simulink model "cddDcmApp"  (R2022b)
settings: Solver=FixedStepDiscrete  StartTime=0.0  StopTime=10.0  FixedStep=0.02  RelTol=1e-3
undefined symbols (not defined in the file or in its folder): CCPFaultType, DcChlType, HFAChlType, IcuChannelType, MtrRoutStaEnum, P_SignalPartType, P_Std_ReturnType, PowerChlType, SrsAdChlType, VGAChlType
(part 11 of 13)

== system cddDcmApp/DataServices_DID_D970_Stepper_Motor_12_Mode_Panel_First_Left_Flap_DID_D970_1_step_Range/DataServices_DID_D970_Stepper_Motor_12_Mode_Panel_First_Left_Flap_DID_D970_1_step_Range_WriteData_sys ==
  function : TriggerPort  TriggerType=function-call  IsSimulinkFunction=on  FunctionName=DataServices_DID_D970_Stepper_Motor_12_Mode_Panel_First_Left_Flap_DID_D970_1_step_Range_WriteData  VariantControl=(inherit)
  Ground_1 : Ground
  Ground_2 : Ground
  Terminator_1 : Terminator
  Terminator_2 : Terminator
  Data : ArgIn  OutDataTypeStr=uint8  PortDimensions=2  ArgumentName=Data
  OpStatus : ArgIn  Port=2  OutDataTypeStr=Enum: Dcm_OpStatusType  ArgumentName=OpStatus
  ErrorCode : ArgOut  OutDataTypeStr=Enum: Dcm_NegativeResponseCodeType  ArgumentName=ErrorCode
  ERR : ArgOut  Port=2  OutDataTypeStr=Std_ReturnType  ArgumentName=ERR
  wires:
  Data/1 -> Terminator_1/1
  OpStatus/1 -> Terminator_2/1
  Ground_1/1 -> ErrorCode/1
  Ground_2/1 -> ERR/1

== system cddDcmApp/DataServices_DID_D970_Stepper_Motor_12_Mode_Panel_First_Left_Flap_DID_D970_2_No_Of_Movements/DataServices_DID_D970_Stepper_Motor_12_Mode_Panel_First_Left_Flap_DID_D970_2_No_Of_Movements_ConditionCheckRead_sys ==
  function : TriggerPort  TriggerType=function-call  IsSimulinkFunction=on  FunctionName=DataServices_DID_D970_Stepper_Motor_12_Mode_Panel_First_Left_Flap_DID_D970_2_No_Of_Movements_ConditionCheckRead  VariantControl=(inherit)
  Ground_1 : Ground
  Ground_2 : Ground
  ErrorCode : ArgOut  OutDataTypeStr=Enum: Dcm_NegativeResponseCodeType  ArgumentName=ErrorCode
  ERR : ArgOut  Port=2  OutDataTypeStr=Std_ReturnType  ArgumentName=ERR
  wires:
  Ground_1/1 -> ErrorCode/1
  Ground_2/1 -> ERR/1

== system cddDcmApp/DataServices_DID_D970_Stepper_Motor_12_Mode_Panel_First_Left_Flap_DID_D970_2_No_Of_Movements/DataServices_DID_D970_Stepper_Motor_12_Mode_Panel_First_Left_Flap_DID_D970_2_No_Of_Movements_ReadData_sys ==
  function : TriggerPort  TriggerType=function-call  IsSimulinkFunction=on  FunctionName=DataServices_DID_D970_Stepper_Motor_12_Mode_Panel_First_Left_Flap_DID_D970_2_No_Of_Movements_ReadData  VariantControl=(inherit)
  Ground_1 : Ground
  Ground_2 : Ground
  Data : ArgOut  OutDataTypeStr=uint8  ArgumentName=Data
  ERR : ArgOut  Port=2  OutDataTypeStr=Std_ReturnType  ArgumentName=ERR
  wires:
  Ground_1/1 -> Data/1
  Ground_2/1 -> ERR/1

== system cddDcmApp/DataServices_DID_D970_Stepper_Motor_12_Mode_Panel_First_Left_Flap_DID_D970_2_No_Of_Movements/DataServices_DID_D970_Stepper_Motor_12_Mode_Panel_First_Left_Flap_DID_D970_2_No_Of_Movements_WriteData_sys ==
  function : TriggerPort  TriggerType=function-call  IsSimulinkFunction=on  FunctionName=DataServices_DID_D970_Stepper_Motor_12_Mode_Panel_First_Left_Flap_DID_D970_2_No_Of_Movements_WriteData  VariantControl=(inherit)
  Ground_1 : Ground
  Ground_2 : Ground
  Terminator_1 : Terminator
  Terminator_2 : Terminator
  Data : ArgIn  OutDataTypeStr=uint8  PortDimensions=4  ArgumentName=Data
  OpStatus : ArgIn  Port=2  OutDataTypeStr=Enum: Dcm_OpStatusType  ArgumentName=OpStatus
  ErrorCode : ArgOut  OutDataTypeStr=Enum: Dcm_NegativeResponseCodeType  ArgumentName=ErrorCode
  ERR : ArgOut  Port=2  OutDataTypeStr=Std_ReturnType  ArgumentName=ERR
  wires:
  Data/1 -> Terminator_1/1
  OpStatus/1 -> Terminator_2/1
  Ground_1/1 -> ErrorCode/1
  Ground_2/1 -> ERR/1

== system cddDcmApp/DataServices_DID_D971_Stepper_Motor_12_Mode_Panel_First_Left_Flap_position_ComplexStructure_2/DataServices_DID_D971_Stepper_Motor_12_Mode_Panel_First_Left_Flap_position_ComplexStructure_2_ConditionCheckRead_sys ==
  function : TriggerPort  TriggerType=function-call  IsSimulinkFunction=on  FunctionName=DataServices_DID_D971_Stepper_Motor_12_Mode_Panel_First_Left_Flap_position_ComplexStructure_2_ConditionCheckRead  VariantControl=(inherit)
  Ground_1 : Ground
  Ground_2 : Ground
  ErrorCode : ArgOut  OutDataTypeStr=Enum: Dcm_NegativeResponseCodeType  ArgumentName=ErrorCode
  ERR : ArgOut  Port=2  OutDataTypeStr=Std_ReturnType  ArgumentName=ERR
  wires:
  Ground_1/1 -> ErrorCode/1
  Ground_2/1 -> ERR/1

== system cddDcmApp/DataServices_DID_D971_Stepper_Motor_12_Mode_Panel_First_Left_Flap_position_ComplexStructure_2/DataServices_DID_D971_Stepper_Motor_12_Mode_Panel_First_Left_Flap_position_ComplexStructure_2_ReadData_sys ==
  function : TriggerPort  TriggerType=function-call  IsSimulinkFunction=on  FunctionName=DataServices_DID_D971_Stepper_Motor_12_Mode_Panel_First_Left_Flap_position_ComplexStructure_2_ReadData  VariantControl=(inherit)
  Ground_1 : Ground
  Ground_2 : Ground
  Data : ArgOut  OutDataTypeStr=uint8  ArgumentName=Data
  ERR : ArgOut  Port=2  OutDataTypeStr=Std_ReturnType  ArgumentName=ERR
  wires:
  Ground_1/1 -> Data/1
  Ground_2/1 -> ERR/1

== system cddDcmApp/DataServices_DID_D971_Stepper_Motor_12_Mode_Panel_First_Left_Flap_position_DID_D971_1_Requested_Position/DataServices_DID_D971_Stepper_Motor_12_Mode_Panel_First_Left_Flap_position_DID_D971_1_Requested_Position_ConditionCheckRead_sys ==
  function : TriggerPort  TriggerType=function-call  IsSimulinkFunction=on  FunctionName=<binary blob, 123 chars, not shown>  VariantControl=(inherit)
  Ground_1 : Ground
  Ground_2 : Ground
  ErrorCode : ArgOut  OutDataTypeStr=Enum: Dcm_NegativeResponseCodeType  ArgumentName=ErrorCode
  ERR : ArgOut  Port=2  OutDataTypeStr=Std_ReturnType  ArgumentName=ERR
  wires:
  Ground_1/1 -> ErrorCode/1
  Ground_2/1 -> ERR/1

== system cddDcmApp/DataServices_DID_D971_Stepper_Motor_12_Mode_Panel_First_Left_Flap_position_DID_D971_1_Requested_Position/DataServices_DID_D971_Stepper_Motor_12_Mode_Panel_First_Left_Flap_position_DID_D971_1_Requested_Position_ReadData_sys ==
  function : TriggerPort  TriggerType=function-call  IsSimulinkFunction=on  FunctionName=DataServices_DID_D971_Stepper_Motor_12_Mode_Panel_First_Left_Flap_position_DID_D971_1_Requested_Position_ReadData  VariantControl=(inherit)
  Ground_1 : Ground
  Ground_2 : Ground
  Data : ArgOut  OutDataTypeStr=uint8  ArgumentName=Data
  ERR : ArgOut  Port=2  OutDataTypeStr=Std_ReturnType  ArgumentName=ERR
  wires:
  Ground_1/1 -> Data/1
  Ground_2/1 -> ERR/1

== system cddDcmApp/DataServices_DID_D971_Stepper_Motor_12_Mode_Panel_First_Left_Flap_position_DID_D971_2_Actual_Position/DataServices_DID_D971_Stepper_Motor_12_Mode_Panel_First_Left_Flap_position_DID_D971_2_Actual_Position_ConditionCheckRead_sys ==
  function : TriggerPort  TriggerType=function-call  IsSimulinkFunction=on  FunctionName=<binary blob, 120 chars, not shown>  VariantControl=(inherit)
  Ground_1 : Ground
  Ground_2 : Ground
  ErrorCode : ArgOut  OutDataTypeStr=Enum: Dcm_NegativeResponseCodeType  ArgumentName=ErrorCode
  ERR : ArgOut  Port=2  OutDataTypeStr=Std_ReturnType  ArgumentName=ERR
  wires:
  Ground_1/1 -> ErrorCode/1
  Ground_2/1 -> ERR/1

== system cddDcmApp/DataServices_DID_D971_Stepper_Motor_12_Mode_Panel_First_Left_Flap_position_DID_D971_2_Actual_Position/DataServices_DID_D971_Stepper_Motor_12_Mode_Panel_First_Left_Flap_position_DID_D971_2_Actual_Position_ReadData_sys ==
  function : TriggerPort  TriggerType=function-call  IsSimulinkFunction=on  FunctionName=DataServices_DID_D971_Stepper_Motor_12_Mode_Panel_First_Left_Flap_position_DID_D971_2_Actual_Position_ReadData  VariantControl=(inherit)
  Ground_1 : Ground
  Ground_2 : Ground
  Data : ArgOut  OutDataTypeStr=uint8  ArgumentName=Data
  ERR : ArgOut  Port=2  OutDataTypeStr=Std_ReturnType  ArgumentName=ERR
  wires:
  Ground_1/1 -> Data/1
  Ground_2/1 -> ERR/1

== system cddDcmApp/DataServices_DID_D972_Stepper_Motor_13_Mode_Panel_First_Side_Left_Flap_DID_D972_1_step_Range/DataServices_DID_D972_Stepper_Motor_13_Mode_Panel_First_Side_Left_Flap_DID_D972_1_step_Range_ConditionCheckRead_sys ==
  function : TriggerPort  TriggerType=function-call  IsSimulinkFunction=on  FunctionName=DataServices_DID_D972_Stepper_Motor_13_Mode_Panel_First_Side_Left_Flap_DID_D972_1_step_Range_ConditionCheckRead  VariantControl=(inherit)
  Ground_1 : Ground
  Ground_2 : Ground
  ErrorCode : ArgOut  OutDataTypeStr=Enum: Dcm_NegativeResponseCodeType  ArgumentName=ErrorCode
  ERR : ArgOut  Port=2  OutDataTypeStr=Std_ReturnType  ArgumentName=ERR
  wires:
  Ground_1/1 -> ErrorCode/1
  Ground_2/1 -> ERR/1

== system cddDcmApp/DataServices_DID_D972_Stepper_Motor_13_Mode_Panel_First_Side_Left_Flap_DID_D972_1_step_Range/DataServices_DID_D972_Stepper_Motor_13_Mode_Panel_First_Side_Left_Flap_DID_D972_1_step_Range_ReadData_sys ==
  function : TriggerPort  TriggerType=function-call  IsSimulinkFunction=on  FunctionName=DataServices_DID_D972_Stepper_Motor_13_Mode_Panel_First_Side_Left_Flap_DID_D972_1_step_Range_ReadData  VariantControl=(inherit)
  Ground_1 : Ground
  Ground_2 : Ground
  Data : ArgOut  OutDataTypeStr=uint8  ArgumentName=Data
  ERR : ArgOut  Port=2  OutDataTypeStr=Std_ReturnType  ArgumentName=ERR
  wires:
  Ground_1/1 -> Data/1
  Ground_2/1 -> ERR/1

== system cddDcmApp/DataServices_DID_D972_Stepper_Motor_13_Mode_Panel_First_Side_Left_Flap_DID_D972_1_step_Range/DataServices_DID_D972_Stepper_Motor_13_Mode_Panel_First_Side_Left_Flap_DID_D972_1_step_Range_WriteData_sys ==
  function : TriggerPort  TriggerType=function-call  IsSimulinkFunction=on  FunctionName=DataServices_DID_D972_Stepper_Motor_13_Mode_Panel_First_Side_Left_Flap_DID_D972_1_step_Range_WriteData  VariantControl=(inherit)
  Ground_1 : Ground
  Ground_2 : Ground
  Terminator_1 : Terminator
  Terminator_2 : Terminator
  Data : ArgIn  OutDataTypeStr=uint8  PortDimensions=2  ArgumentName=Data
  OpStatus : ArgIn  Port=2  OutDataTypeStr=Enum: Dcm_OpStatusType  ArgumentName=OpStatus
  ErrorCode : ArgOut  OutDataTypeStr=Enum: Dcm_NegativeResponseCodeType  ArgumentName=ErrorCode
  ERR : ArgOut  Port=2  OutDataTypeStr=Std_ReturnType  ArgumentName=ERR
  wires:
  Data/1 -> Terminator_1/1
  OpStatus/1 -> Terminator_2/1
  Ground_1/1 -> ErrorCode/1
  Ground_2/1 -> ERR/1

== system cddDcmApp/DataServices_DID_D972_Stepper_Motor_13_Mode_Panel_First_Side_Left_Flap_DID_D972_2_No_Of_Movements/DataServices_DID_D972_Stepper_Motor_13_Mode_Panel_First_Side_Left_Flap_DID_D972_2_No_Of_Movements_ConditionCheckRead_sys ==
  function : TriggerPort  TriggerType=function-call  IsSimulinkFunction=on  FunctionName=DataServices_DID_D972_Stepper_Motor_13_Mode_Panel_First_Side_Left_Flap_DID_D972_2_No_Of_Movements_ConditionCheckRead  VariantControl=(inherit)
  Ground_1 : Ground
  Ground_2 : Ground
  ErrorCode : ArgOut  OutDataTypeStr=Enum: Dcm_NegativeResponseCodeType  ArgumentName=ErrorCode
  ERR : ArgOut  Port=2  OutDataTypeStr=Std_ReturnType  ArgumentName=ERR
  wires:
  Ground_1/1 -> ErrorCode/1
  Ground_2/1 -> ERR/1

== system cddDcmApp/DataServices_DID_D972_Stepper_Motor_13_Mode_Panel_First_Side_Left_Flap_DID_D972_2_No_Of_Movements/DataServices_DID_D972_Stepper_Motor_13_Mode_Panel_First_Side_Left_Flap_DID_D972_2_No_Of_Movements_ReadData_sys ==
  function : TriggerPort  TriggerType=function-call  IsSimulinkFunction=on  FunctionName=DataServices_DID_D972_Stepper_Motor_13_Mode_Panel_First_Side_Left_Flap_DID_D972_2_No_Of_Movements_ReadData  VariantControl=(inherit)
  Ground_1 : Ground
  Ground_2 : Ground
  Data : ArgOut  OutDataTypeStr=uint8  ArgumentName=Data
  ERR : ArgOut  Port=2  OutDataTypeStr=Std_ReturnType  ArgumentName=ERR
  wires:
  Ground_1/1 -> Data/1
  Ground_2/1 -> ERR/1

== system cddDcmApp/DataServices_DID_D972_Stepper_Motor_13_Mode_Panel_First_Side_Left_Flap_DID_D972_2_No_Of_Movements/DataServices_DID_D972_Stepper_Motor_13_Mode_Panel_First_Side_Left_Flap_DID_D972_2_No_Of_Movements_WriteData_sys ==
  function : TriggerPort  TriggerType=function-call  IsSimulinkFunction=on  FunctionName=DataServices_DID_D972_Stepper_Motor_13_Mode_Panel_First_Side_Left_Flap_DID_D972_2_No_Of_Movements_WriteData  VariantControl=(inherit)
  Ground_1 : Ground
  Ground_2 : Ground
  Terminator_1 : Terminator
  Terminator_2 : Terminator
  Data : ArgIn  OutDataTypeStr=uint8  PortDimensions=4  ArgumentName=Data
  OpStatus : ArgIn  Port=2  OutDataTypeStr=Enum: Dcm_OpStatusType  ArgumentName=OpStatus
  ErrorCode : ArgOut  OutDataTypeStr=Enum: Dcm_NegativeResponseCodeType  ArgumentName=ErrorCode
  ERR : ArgOut  Port=2  OutDataTypeStr=Std_ReturnType  ArgumentName=ERR
  wires:
  Data/1 -> Terminator_1/1
  OpStatus/1 -> Terminator_2/1
  Ground_1/1 -> ErrorCode/1
  Ground_2/1 -> ERR/1

== system cddDcmApp/DataServices_DID_D973_Stepper_Motor_13_Mode_Panel_First_Side_Left_Flap_position_ComplexStructure_2/DataServices_DID_D973_Stepper_Motor_13_Mode_Panel_First_Side_Left_Flap_position_ComplexStructure_2_ConditionCheckRead_sys ==
  function : TriggerPort  TriggerType=function-call  IsSimulinkFunction=on  FunctionName=DataServices_DID_D973_Stepper_Motor_13_Mode_Panel_First_Side_Left_Flap_position_ComplexStructure_2_ConditionCheckRead  VariantControl=(inherit)
  Ground_1 : Ground
  Ground_2 : Ground
  ErrorCode : ArgOut  OutDataTypeStr=Enum: Dcm_NegativeResponseCodeType  ArgumentName=ErrorCode
  ERR : ArgOut  Port=2  OutDataTypeStr=Std_ReturnType  ArgumentName=ERR
  wires:
  Ground_1/1 -> ErrorCode/1
  Ground_2/1 -> ERR/1

== system cddDcmApp/DataServices_DID_D973_Stepper_Motor_13_Mode_Panel_First_Side_Left_Flap_position_ComplexStructure_2/DataServices_DID_D973_Stepper_Motor_13_Mode_Panel_First_Side_Left_Flap_position_ComplexStructure_2_ReadData_sys ==
  function : TriggerPort  TriggerType=function-call  IsSimulinkFunction=on  FunctionName=DataServices_DID_D973_Stepper_Motor_13_Mode_Panel_First_Side_Left_Flap_position_ComplexStructure_2_ReadData  VariantControl=(inherit)
  Ground_1 : Ground
  Ground_2 : Ground
  Data : ArgOut  OutDataTypeStr=uint8  ArgumentName=Data
  ERR : ArgOut  Port=2  OutDataTypeStr=Std_ReturnType  ArgumentName=ERR
  wires:
  Ground_1/1 -> Data/1
  Ground_2/1 -> ERR/1

== system cddDcmApp/DataServices_DID_D973_Stepper_Motor_13_Mode_Panel_First_Side_Left_Flap_position_DID_D973_1_Requested_Position/DataServices_DID_D973_Stepper_Motor_13_Mode_Panel_First_Side_Left_Flap_position_DID_D973_1_Requested_Position_ConditionCheckRead_sys ==
  function : TriggerPort  TriggerType=function-call  IsSimulinkFunction=on  FunctionName=<binary blob, 128 chars, not shown>  VariantControl=(inherit)
  Ground_1 : Ground
  Ground_2 : Ground
  ErrorCode : ArgOut  OutDataTypeStr=Enum: Dcm_NegativeResponseCodeType  ArgumentName=ErrorCode
  ERR : ArgOut  Port=2  OutDataTypeStr=Std_ReturnType  ArgumentName=ERR
  wires:
  Ground_1/1 -> ErrorCode/1
  Ground_2/1 -> ERR/1

== system cddDcmApp/DataServices_DID_D973_Stepper_Motor_13_Mode_Panel_First_Side_Left_Flap_position_DID_D973_1_Requested_Position/DataServices_DID_D973_Stepper_Motor_13_Mode_Panel_First_Side_Left_Flap_position_DID_D973_1_Requested_Position_ReadData_sys ==
  function : TriggerPort  TriggerType=function-call  IsSimulinkFunction=on  FunctionName=DataServices_DID_D973_Stepper_Motor_13_Mode_Panel_First_Side_Left_Flap_position_DID_D973_1_Requested_Position_ReadData  VariantControl=(inherit)
  Ground_1 : Ground
  Ground_2 : Ground
  Data : ArgOut  OutDataTypeStr=uint8  ArgumentName=Data
  ERR : ArgOut  Port=2  OutDataTypeStr=Std_ReturnType  ArgumentName=ERR
  wires:
  Ground_1/1 -> Data/1
  Ground_2/1 -> ERR/1

== system cddDcmApp/DataServices_DID_D973_Stepper_Motor_13_Mode_Panel_First_Side_Left_Flap_position_DID_D973_2_Actual_Position/DataServices_DID_D973_Stepper_Motor_13_Mode_Panel_First_Side_Left_Flap_position_DID_D973_2_Actual_Position_ConditionCheckRead_sys ==
  function : TriggerPort  TriggerType=function-call  IsSimulinkFunction=on  FunctionName=<binary blob, 125 chars, not shown>  VariantControl=(inherit)
  Ground_1 : Ground
  Ground_2 : Ground
  ErrorCode : ArgOut  OutDataTypeStr=Enum: Dcm_NegativeResponseCodeType  ArgumentName=ErrorCode
  ERR : ArgOut  Port=2  OutDataTypeStr=Std_ReturnType  ArgumentName=ERR
  wires:
  Ground_1/1 -> ErrorCode/1
  Ground_2/1 -> ERR/1

== system cddDcmApp/DataServices_DID_D973_Stepper_Motor_13_Mode_Panel_First_Side_Left_Flap_position_DID_D973_2_Actual_Position/DataServices_DID_D973_Stepper_Motor_13_Mode_Panel_First_Side_Left_Flap_position_DID_D973_2_Actual_Position_ReadData_sys ==
  function : TriggerPort  TriggerType=function-call  IsSimulinkFunction=on  FunctionName=DataServices_DID_D973_Stepper_Motor_13_Mode_Panel_First_Side_Left_Flap_position_DID_D973_2_Actual_Position_ReadData  VariantControl=(inherit)
  Ground_1 : Ground
  Ground_2 : Ground
  Data : ArgOut  OutDataTypeStr=uint8  ArgumentName=Data
  ERR : ArgOut  Port=2  OutDataTypeStr=Std_ReturnType  ArgumentName=ERR
  wires:
  Ground_1/1 -> Data/1
  Ground_2/1 -> ERR/1

== system cddDcmApp/DataServices_DID_D974_Stepper_Motor_14_Mode_Panel_First_Right_Flap_DID_D974_1_step_Range/DataServices_DID_D974_Stepper_Motor_14_Mode_Panel_First_Right_Flap_DID_D974_1_step_Range_ConditionCheckRead_sys ==
  function : TriggerPort  TriggerType=function-call  IsSimulinkFunction=on  FunctionName=DataServices_DID_D974_Stepper_Motor_14_Mode_Panel_First_Right_Flap_DID_D974_1_step_Range_ConditionCheckRead  VariantControl=(inherit)
  Ground_1 : Ground
  Ground_2 : Ground
  ErrorCode : ArgOut  OutDataTypeStr=Enum: Dcm_NegativeResponseCodeType  ArgumentName=ErrorCode
  ERR : ArgOut  Port=2  OutDataTypeStr=Std_ReturnType  ArgumentName=ERR
  wires:
  Ground_1/1 -> ErrorCode/1
  Ground_2/1 -> ERR/1

== system cddDcmApp/DataServices_DID_D974_Stepper_Motor_14_Mode_Panel_First_Right_Flap_DID_D974_1_step_Range/DataServices_DID_D974_Stepper_Motor_14_Mode_Panel_First_Right_Flap_DID_D974_1_step_Range_ReadData_sys ==
  function : TriggerPort  TriggerType=function-call  IsSimulinkFunction=on  FunctionName=DataServices_DID_D974_Stepper_Motor_14_Mode_Panel_First_Right_Flap_DID_D974_1_step_Range_ReadData  VariantControl=(inherit)
  Ground_1 : Ground
  Ground_2 : Ground
  Data : ArgOut  OutDataTypeStr=uint8  ArgumentName=Data
  ERR : ArgOut  Port=2  OutDataTypeStr=Std_ReturnType  ArgumentName=ERR
  wires:
  Ground_1/1 -> Data/1
  Ground_2/1 -> ERR/1

== system cddDcmApp/DataServices_DID_D974_Stepper_Motor_14_Mode_Panel_First_Right_Flap_DID_D974_1_step_Range/DataServices_DID_D974_Stepper_Motor_14_Mode_Panel_First_Right_Flap_DID_D974_1_step_Range_WriteData_sys ==
  function : TriggerPort  TriggerType=function-call  IsSimulinkFunction=on  FunctionName=DataServices_DID_D974_Stepper_Motor_14_Mode_Panel_First_Right_Flap_DID_D974_1_step_Range_WriteData  VariantControl=(inherit)
  Ground_1 : Ground
  Ground_2 : Ground
  Terminator_1 : Terminator
  Terminator_2 : Terminator
  Data : ArgIn  OutDataTypeStr=uint8  PortDimensions=2  ArgumentName=Data
  OpStatus : ArgIn  Port=2  OutDataTypeStr=Enum: Dcm_OpStatusType  ArgumentName=OpStatus
  ErrorCode : ArgOut  OutDataTypeStr=Enum: Dcm_NegativeResponseCodeType  ArgumentName=ErrorCode
  ERR : ArgOut  Port=2  OutDataTypeStr=Std_ReturnType  ArgumentName=ERR
  wires:
  Data/1 -> Terminator_1/1
  OpStatus/1 -> Terminator_2/1
  Ground_1/1 -> ErrorCode/1
  Ground_2/1 -> ERR/1

== system cddDcmApp/DataServices_DID_D974_Stepper_Motor_14_Mode_Panel_First_Right_Flap_DID_D974_2_No_Of_Movements/DataServices_DID_D974_Stepper_Motor_14_Mode_Panel_First_Right_Flap_DID_D974_2_No_Of_Movements_ConditionCheckRead_sys ==
  function : TriggerPort  TriggerType=function-call  IsSimulinkFunction=on  FunctionName=DataServices_DID_D974_Stepper_Motor_14_Mode_Panel_First_Right_Flap_DID_D974_2_No_Of_Movements_ConditionCheckRead  VariantControl=(inherit)
  Ground_1 : Ground
  Ground_2 : Ground
  ErrorCode : ArgOut  OutDataTypeStr=Enum: Dcm_NegativeResponseCodeType  ArgumentName=ErrorCode
  ERR : ArgOut  Port=2  OutDataTypeStr=Std_ReturnType  ArgumentName=ERR
  wires:
  Ground_1/1 -> ErrorCode/1
  Ground_2/1 -> ERR/1

== system cddDcmApp/DataServices_DID_D974_Stepper_Motor_14_Mode_Panel_First_Right_Flap_DID_D974_2_No_Of_Movements/DataServices_DID_D974_Stepper_Motor_14_Mode_Panel_First_Right_Flap_DID_D974_2_No_Of_Movements_ReadData_sys ==
  function : TriggerPort  TriggerType=function-call  IsSimulinkFunction=on  FunctionName=DataServices_DID_D974_Stepper_Motor_14_Mode_Panel_First_Right_Flap_DID_D974_2_No_Of_Movements_ReadData  VariantControl=(inherit)
  Ground_1 : Ground
  Ground_2 : Ground
  Data : ArgOut  OutDataTypeStr=uint8  ArgumentName=Data
  ERR : ArgOut  Port=2  OutDataTypeStr=Std_ReturnType  ArgumentName=ERR
  wires:
  Ground_1/1 -> Data/1
  Ground_2/1 -> ERR/1

== system cddDcmApp/DataServices_DID_D974_Stepper_Motor_14_Mode_Panel_First_Right_Flap_DID_D974_2_No_Of_Movements/DataServices_DID_D974_Stepper_Motor_14_Mode_Panel_First_Right_Flap_DID_D974_2_No_Of_Movements_WriteData_sys ==
  function : TriggerPort  TriggerType=function-call  IsSimulinkFunction=on  FunctionName=DataServices_DID_D974_Stepper_Motor_14_Mode_Panel_First_Right_Flap_DID_D974_2_No_Of_Movements_WriteData  VariantControl=(inherit)
  Ground_1 : Ground
  Ground_2 : Ground
  Terminator_1 : Terminator
  Terminator_2 : Terminator
  Data : ArgIn  OutDataTypeStr=uint8  PortDimensions=4  ArgumentName=Data
  OpStatus : ArgIn  Port=2  OutDataTypeStr=Enum: Dcm_OpStatusType  ArgumentName=OpStatus
  ErrorCode : ArgOut  OutDataTypeStr=Enum: Dcm_NegativeResponseCodeType  ArgumentName=ErrorCode
  ERR : ArgOut  Port=2  OutDataTypeStr=Std_ReturnType  ArgumentName=ERR
  wires:
  Data/1 -> Terminator_1/1
  OpStatus/1 -> Terminator_2/1
  Ground_1/1 -> ErrorCode/1
  Ground_2/1 -> ERR/1

== system cddDcmApp/DataServices_DID_D975_Stepper_Motor_14_Mode_Panel_First_Right_Flap_position_ComplexStructure_2/DataServices_DID_D975_Stepper_Motor_14_Mode_Panel_First_Right_Flap_position_ComplexStructure_2_ConditionCheckRead_sys ==
  function : TriggerPort  TriggerType=function-call  IsSimulinkFunction=on  FunctionName=DataServices_DID_D975_Stepper_Motor_14_Mode_Panel_First_Right_Flap_position_ComplexStructure_2_ConditionCheckRead  VariantControl=(inherit)
  Ground_1 : Ground
  Ground_2 : Ground
  ErrorCode : ArgOut  OutDataTypeStr=Enum: Dcm_NegativeResponseCodeType  ArgumentName=ErrorCode
  ERR : ArgOut  Port=2  OutDataTypeStr=Std_ReturnType  ArgumentName=ERR
  wires:
  Ground_1/1 -> ErrorCode/1
  Ground_2/1 -> ERR/1

== system cddDcmApp/DataServices_DID_D975_Stepper_Motor_14_Mode_Panel_First_Right_Flap_position_ComplexStructure_2/DataServices_DID_D975_Stepper_Motor_14_Mode_Panel_First_Right_Flap_position_ComplexStructure_2_ReadData_sys ==
  function : TriggerPort  TriggerType=function-call  IsSimulinkFunction=on  FunctionName=DataServices_DID_D975_Stepper_Motor_14_Mode_Panel_First_Right_Flap_position_ComplexStructure_2_ReadData  VariantControl=(inherit)
  Ground_1 : Ground
  Ground_2 : Ground
  Data : ArgOut  OutDataTypeStr=uint8  ArgumentName=Data
  ERR : ArgOut  Port=2  OutDataTypeStr=Std_ReturnType  ArgumentName=ERR
  wires:
  Ground_1/1 -> Data/1
  Ground_2/1 -> ERR/1

== system cddDcmApp/DataServices_DID_D975_Stepper_Motor_14_Mode_Panel_First_Right_Flap_position_DID_D975_1_Requested_Position/DataServices_DID_D975_Stepper_Motor_14_Mode_Panel_First_Right_Flap_position_DID_D975_1_Requested_Position_ConditionCheckRead_sys ==
  function : TriggerPort  TriggerType=function-call  IsSimulinkFunction=on  FunctionName=<binary blob, 124 chars, not shown>  VariantControl=(inherit)
  Ground_1 : Ground
  Ground_2 : Ground
  ErrorCode : ArgOut  OutDataTypeStr=Enum: Dcm_NegativeResponseCodeType  ArgumentName=ErrorCode
  ERR : ArgOut  Port=2  OutDataTypeStr=Std_ReturnType  ArgumentName=ERR
  wires:
  Ground_1/1 -> ErrorCode/1
  Ground_2/1 -> ERR/1

== system cddDcmApp/DataServices_DID_D975_Stepper_Motor_14_Mode_Panel_First_Right_Flap_position_DID_D975_1_Requested_Position/DataServices_DID_D975_Stepper_Motor_14_Mode_Panel_First_Right_Flap_position_DID_D975_1_Requested_Position_ReadData_sys ==
  function : TriggerPort  TriggerType=function-call  IsSimulinkFunction=on  FunctionName=DataServices_DID_D975_Stepper_Motor_14_Mode_Panel_First_Right_Flap_position_DID_D975_1_Requested_Position_ReadData  VariantControl=(inherit)
  Ground_1 : Ground
  Ground_2 : Ground
  Data : ArgOut  OutDataTypeStr=uint8  ArgumentName=Data
  ERR : ArgOut  Port=2  OutDataTypeStr=Std_ReturnType  ArgumentName=ERR
  wires:
  Ground_1/1 -> Data/1
  Ground_2/1 -> ERR/1

== system cddDcmApp/DataServices_DID_D975_Stepper_Motor_14_Mode_Panel_First_Right_Flap_position_DID_D975_2_Actual_Position/DataServices_DID_D975_Stepper_Motor_14_Mode_Panel_First_Right_Flap_position_DID_D975_2_Actual_Position_ConditionCheckRead_sys ==
  function : TriggerPort  TriggerType=function-call  IsSimulinkFunction=on  FunctionName=<binary blob, 121 chars, not shown>  VariantControl=(inherit)
  Ground_1 : Ground
  Ground_2 : Ground
  ErrorCode : ArgOut  OutDataTypeStr=Enum: Dcm_NegativeResponseCodeType  ArgumentName=ErrorCode
  ERR : ArgOut  Port=2  OutDataTypeStr=Std_ReturnType  ArgumentName=ERR
  wires:
  Ground_1/1 -> ErrorCode/1
  Ground_2/1 -> ERR/1

== system cddDcmApp/DataServices_DID_D975_Stepper_Motor_14_Mode_Panel_First_Right_Flap_position_DID_D975_2_Actual_Position/DataServices_DID_D975_Stepper_Motor_14_Mode_Panel_First_Right_Flap_position_DID_D975_2_Actual_Position_ReadData_sys ==
  function : TriggerPort  TriggerType=function-call  IsSimulinkFunction=on  FunctionName=DataServices_DID_D975_Stepper_Motor_14_Mode_Panel_First_Right_Flap_position_DID_D975_2_Actual_Position_ReadData  VariantControl=(inherit)
  Ground_1 : Ground
  Ground_2 : Ground
  Data : ArgOut  OutDataTypeStr=uint8  ArgumentName=Data
  ERR : ArgOut  Port=2  OutDataTypeStr=Std_ReturnType  ArgumentName=ERR
  wires:
  Ground_1/1 -> Data/1
  Ground_2/1 -> ERR/1

== system cddDcmApp/DataServices_DID_D976_Stepper_Motor_15_Mode_Panel_First_Side_Right_Flap_DID_D976_1_step_Range/DataServices_DID_D976_Stepper_Motor_15_Mode_Panel_First_Side_Right_Flap_DID_D976_1_step_Range_ConditionCheckRead_sys ==
  function : TriggerPort  TriggerType=function-call  IsSimulinkFunction=on  FunctionName=DataServices_DID_D976_Stepper_Motor_15_Mode_Panel_First_Side_Right_Flap_DID_D976_1_step_Range_ConditionCheckRead  VariantControl=(inherit)
  Ground_1 : Ground
  Ground_2 : Ground
  ErrorCode : ArgOut  OutDataTypeStr=Enum: Dcm_NegativeResponseCodeType  ArgumentName=ErrorCode
  ERR : ArgOut  Port=2  OutDataTypeStr=Std_ReturnType  ArgumentName=ERR
  wires:
  Ground_1/1 -> ErrorCode/1
  Ground_2/1 -> ERR/1

== system cddDcmApp/DataServices_DID_D976_Stepper_Motor_15_Mode_Panel_First_Side_Right_Flap_DID_D976_1_step_Range/DataServices_DID_D976_Stepper_Motor_15_Mode_Panel_First_Side_Right_Flap_DID_D976_1_step_Range_ReadData_sys ==
  function : TriggerPort  TriggerType=function-call  IsSimulinkFunction=on  FunctionName=DataServices_DID_D976_Stepper_Motor_15_Mode_Panel_First_Side_Right_Flap_DID_D976_1_step_Range_ReadData  VariantControl=(inherit)
  Ground_1 : Ground
  Ground_2 : Ground
  Data : ArgOut  OutDataTypeStr=uint8  ArgumentName=Data
  ERR : ArgOut  Port=2  OutDataTypeStr=Std_ReturnType  ArgumentName=ERR
  wires:
  Ground_1/1 -> Data/1
  Ground_2/1 -> ERR/1

== system cddDcmApp/DataServices_DID_D976_Stepper_Motor_15_Mode_Panel_First_Side_Right_Flap_DID_D976_1_step_Range/DataServices_DID_D976_Stepper_Motor_15_Mode_Panel_First_Side_Right_Flap_DID_D976_1_step_Range_WriteData_sys ==
  function : TriggerPort  TriggerType=function-call  IsSimulinkFunction=on  FunctionName=DataServices_DID_D976_Stepper_Motor_15_Mode_Panel_First_Side_Right_Flap_DID_D976_1_step_Range_WriteData  VariantControl=(inherit)
  Ground_1 : Ground
  Ground_2 : Ground
  Terminator_1 : Terminator
  Terminator_2 : Terminator
  Data : ArgIn  OutDataTypeStr=uint8  PortDimensions=2  ArgumentName=Data
  OpStatus : ArgIn  Port=2  OutDataTypeStr=Enum: Dcm_OpStatusType  ArgumentName=OpStatus
  ErrorCode : ArgOut  OutDataTypeStr=Enum: Dcm_NegativeResponseCodeType  ArgumentName=ErrorCode
  ERR : ArgOut  Port=2  OutDataTypeStr=Std_ReturnType  ArgumentName=ERR
  wires:
  Data/1 -> Terminator_1/1
  OpStatus/1 -> Terminator_2/1
  Ground_1/1 -> ErrorCode/1
  Ground_2/1 -> ERR/1

== system cddDcmApp/DataServices_DID_D976_Stepper_Motor_15_Mode_Panel_First_Side_Right_Flap_DID_D976_2_No_Of_Movements/DataServices_DID_D976_Stepper_Motor_15_Mode_Panel_First_Side_Right_Flap_DID_D976_2_No_Of_Movements_ConditionCheckRead_sys ==
  function : TriggerPort  TriggerType=function-call  IsSimulinkFunction=on  FunctionName=DataServices_DID_D976_Stepper_Motor_15_Mode_Panel_First_Side_Right_Flap_DID_D976_2_No_Of_Movements_ConditionCheckRead  VariantControl=(inherit)
  Ground_1 : Ground
  Ground_2 : Ground
  ErrorCode : ArgOut  OutDataTypeStr=Enum: Dcm_NegativeResponseCodeType  ArgumentName=ErrorCode
  ERR : ArgOut  Port=2  OutDataTypeStr=Std_ReturnType  ArgumentName=ERR
  wires:
  Ground_1/1 -> ErrorCode/1
  Ground_2/1 -> ERR/1

== system cddDcmApp/DataServices_DID_D976_Stepper_Motor_15_Mode_Panel_First_Side_Right_Flap_DID_D976_2_No_Of_Movements/DataServices_DID_D976_Stepper_Motor_15_Mode_Panel_First_Side_Right_Flap_DID_D976_2_No_Of_Movements_ReadData_sys ==
  function : TriggerPort  TriggerType=function-call  IsSimulinkFunction=on  FunctionName=DataServices_DID_D976_Stepper_Motor_15_Mode_Panel_First_Side_Right_Flap_DID_D976_2_No_Of_Movements_ReadData  VariantControl=(inherit)
  Ground_1 : Ground
  Ground_2 : Ground
  Data : ArgOut  OutDataTypeStr=uint8  ArgumentName=Data
  ERR : ArgOut  Port=2  OutDataTypeStr=Std_ReturnType  ArgumentName=ERR
  wires:
  Ground_1/1 -> Data/1
  Ground_2/1 -> ERR/1

== system cddDcmApp/DataServices_DID_D976_Stepper_Motor_15_Mode_Panel_First_Side_Right_Flap_DID_D976_2_No_Of_Movements/DataServices_DID_D976_Stepper_Motor_15_Mode_Panel_First_Side_Right_Flap_DID_D976_2_No_Of_Movements_WriteData_sys ==
  function : TriggerPort  TriggerType=function-call  IsSimulinkFunction=on  FunctionName=DataServices_DID_D976_Stepper_Motor_15_Mode_Panel_First_Side_Right_Flap_DID_D976_2_No_Of_Movements_WriteData  VariantControl=(inherit)
  Ground_1 : Ground
  Ground_2 : Ground
  Terminator_1 : Terminator
  Terminator_2 : Terminator
  Data : ArgIn  OutDataTypeStr=uint8  PortDimensions=4  ArgumentName=Data
  OpStatus : ArgIn  Port=2  OutDataTypeStr=Enum: Dcm_OpStatusType  ArgumentName=OpStatus
  ErrorCode : ArgOut  OutDataTypeStr=Enum: Dcm_NegativeResponseCodeType  ArgumentName=ErrorCode
  ERR : ArgOut  Port=2  OutDataTypeStr=Std_ReturnType  ArgumentName=ERR
  wires:
  Data/1 -> Terminator_1/1
  OpStatus/1 -> Terminator_2/1
  Ground_1/1 -> ErrorCode/1
  Ground_2/1 -> ERR/1

== system cddDcmApp/DataServices_DID_D977_Stepper_Motor_15_Mode_Panel_First_Side_Right_Flap_position_ComplexStructure_2/DataServices_DID_D977_Stepper_Motor_15_Mode_Panel_First_Side_Right_Flap_position_ComplexStructure_2_ConditionCheckRead_sys ==
  function : TriggerPort  TriggerType=function-call  IsSimulinkFunction=on  FunctionName=DataServices_DID_D977_Stepper_Motor_15_Mode_Panel_First_Side_Right_Flap_position_ComplexStructure_2_ConditionCheckRead  VariantControl=(inherit)
  Ground_1 : Ground
  Ground_2 : Ground
  ErrorCode : ArgOut  OutDataTypeStr=Enum: Dcm_NegativeResponseCodeType  ArgumentName=ErrorCode
  ERR : ArgOut  Port=2  OutDataTypeStr=Std_ReturnType  ArgumentName=ERR
  wires:
  Ground_1/1 -> ErrorCode/1
  Ground_2/1 -> ERR/1

== system cddDcmApp/DataServices_DID_D977_Stepper_Motor_15_Mode_Panel_First_Side_Right_Flap_position_ComplexStructure_2/DataServices_DID_D977_Stepper_Motor_15_Mode_Panel_First_Side_Right_Flap_position_ComplexStructure_2_ReadData_sys ==
  function : TriggerPort  TriggerType=function-call  IsSimulinkFunction=on  FunctionName=DataServices_DID_D977_Stepper_Motor_15_Mode_Panel_First_Side_Right_Flap_position_ComplexStructure_2_ReadData  VariantControl=(inherit)
  Ground_1 : Ground
  Ground_2 : Ground
  Data : ArgOut  OutDataTypeStr=uint8  ArgumentName=Data
  ERR : ArgOut  Port=2  OutDataTypeStr=Std_ReturnType  ArgumentName=ERR
  wires:
  Ground_1/1 -> Data/1
  Ground_2/1 -> ERR/1

== system cddDcmApp/DataServices_DID_D977_Stepper_Motor_15_Mode_Panel_First_Side_Right_Flap_position_DID_D977_1_Requested_Position/DataServices_DID_D977_Stepper_Motor_15_Mode_Panel_First_Side_Right_Flap_position_DID_D977_1_Requested_Position_ReadData_sys ==
  function : TriggerPort  TriggerType=function-call  IsSimulinkFunction=on  FunctionName=DataServices_DID_D977_Stepper_Motor_15_Mode_Panel_First_Side_Right_Flap_position_DID_D977_1_Requested_Position_ReadData  VariantControl=(inherit)
  Ground_1 : Ground
  Ground_2 : Ground
  Data : ArgOut  OutDataTypeStr=uint8  ArgumentName=Data
  ERR : ArgOut  Port=2  OutDataTypeStr=Std_ReturnType  ArgumentName=ERR
  wires:
  Ground_1/1 -> Data/1
  Ground_2/1 -> ERR/1

== system cddDcmApp/DataServices_DID_D977_Stepper_Motor_15_Mode_Panel_First_Side_Right_Flap_position_DID_D977_2_Actual_Position/DataServices_DID_D977_Stepper_Motor_15_Mode_Panel_First_Side_Right_Flap_position_DID_D977_2_Actual_Position_ConditionCheckRead_sys ==
  function : TriggerPort  TriggerType=function-call  IsSimulinkFunction=on  FunctionName=<binary blob, 126 chars, not shown>  VariantControl=(inherit)
  Ground_1 : Ground
  Ground_2 : Ground
  ErrorCode : ArgOut  OutDataTypeStr=Enum: Dcm_NegativeResponseCodeType  ArgumentName=ErrorCode
  ERR : ArgOut  Port=2  OutDataTypeStr=Std_ReturnType  ArgumentName=ERR
  wires:
  Ground_1/1 -> ErrorCode/1
  Ground_2/1 -> ERR/1

== system cddDcmApp/DataServices_DID_D977_Stepper_Motor_15_Mode_Panel_First_Side_Right_Flap_position_DID_D977_2_Actual_Position/DataServices_DID_D977_Stepper_Motor_15_Mode_Panel_First_Side_Right_Flap_position_DID_D977_2_Actual_Position_ReadData_sys ==
  function : TriggerPort  TriggerType=function-call  IsSimulinkFunction=on  FunctionName=DataServices_DID_D977_Stepper_Motor_15_Mode_Panel_First_Side_Right_Flap_position_DID_D977_2_Actual_Position_ReadData  VariantControl=(inherit)
  Ground_1 : Ground
  Ground_2 : Ground
  Data : ArgOut  OutDataTypeStr=uint8  ArgumentName=Data
  ERR : ArgOut  Port=2  OutDataTypeStr=Std_ReturnType  ArgumentName=ERR
  wires:
  Ground_1/1 -> Data/1
  Ground_2/1 -> ERR/1

== system cddDcmApp/DataServices_DID_D978_Stepper_Motor_16_Mode_Floor_First_Left_Flap_DID_D978_1_step_Range/DataServices_DID_D978_Stepper_Motor_16_Mode_Floor_First_Left_Flap_DID_D978_1_step_Range_ConditionCheckRead_sys ==
  function : TriggerPort  TriggerType=function-call  IsSimulinkFunction=on  FunctionName=DataServices_DID_D978_Stepper_Motor_16_Mode_Floor_First_Left_Flap_DID_D978_1_step_Range_ConditionCheckRead  VariantControl=(inherit)
  Ground_1 : Ground
  Ground_2 : Ground
  ErrorCode : ArgOut  OutDataTypeStr=Enum: Dcm_NegativeResponseCodeType  ArgumentName=ErrorCode
  ERR : ArgOut  Port=2  OutDataTypeStr=Std_ReturnType  ArgumentName=ERR
  wires:
  Ground_1/1 -> ErrorCode/1
  Ground_2/1 -> ERR/1

== system cddDcmApp/DataServices_DID_D978_Stepper_Motor_16_Mode_Floor_First_Left_Flap_DID_D978_1_step_Range/DataServices_DID_D978_Stepper_Motor_16_Mode_Floor_First_Left_Flap_DID_D978_1_step_Range_ReadData_sys ==
  function : TriggerPort  TriggerType=function-call  IsSimulinkFunction=on  FunctionName=DataServices_DID_D978_Stepper_Motor_16_Mode_Floor_First_Left_Flap_DID_D978_1_step_Range_ReadData  VariantControl=(inherit)
  Ground_1 : Ground
  Ground_2 : Ground
  Data : ArgOut  OutDataTypeStr=uint8  ArgumentName=Data
  ERR : ArgOut  Port=2  OutDataTypeStr=Std_ReturnType  ArgumentName=ERR
  wires:
  Ground_1/1 -> Data/1
  Ground_2/1 -> ERR/1

== system cddDcmApp/DataServices_DID_D978_Stepper_Motor_16_Mode_Floor_First_Left_Flap_DID_D978_1_step_Range/DataServices_DID_D978_Stepper_Motor_16_Mode_Floor_First_Left_Flap_DID_D978_1_step_Range_WriteData_sys ==
  function : TriggerPort  TriggerType=function-call  IsSimulinkFunction=on  FunctionName=DataServices_DID_D978_Stepper_Motor_16_Mode_Floor_First_Left_Flap_DID_D978_1_step_Range_WriteData  VariantControl=(inherit)
  Ground_1 : Ground
  Ground_2 : Ground
  Terminator_1 : Terminator
  Terminator_2 : Terminator
  Data : ArgIn  OutDataTypeStr=uint8  PortDimensions=2  ArgumentName=Data
  OpStatus : ArgIn  Port=2  OutDataTypeStr=Enum: Dcm_OpStatusType  ArgumentName=OpStatus
  ErrorCode : ArgOut  OutDataTypeStr=Enum: Dcm_NegativeResponseCodeType  ArgumentName=ErrorCode
  ERR : ArgOut  Port=2  OutDataTypeStr=Std_ReturnType  ArgumentName=ERR
  wires:
  Data/1 -> Terminator_1/1
  OpStatus/1 -> Terminator_2/1
  Ground_1/1 -> ErrorCode/1
  Ground_2/1 -> ERR/1

== system cddDcmApp/DataServices_DID_D978_Stepper_Motor_16_Mode_Floor_First_Left_Flap_DID_D978_2_No_Of_Movements/DataServices_DID_D978_Stepper_Motor_16_Mode_Floor_First_Left_Flap_DID_D978_2_No_Of_Movements_ConditionCheckRead_sys ==
  function : TriggerPort  TriggerType=function-call  IsSimulinkFunction=on  FunctionName=DataServices_DID_D978_Stepper_Motor_16_Mode_Floor_First_Left_Flap_DID_D978_2_No_Of_Movements_ConditionCheckRead  VariantControl=(inherit)
  Ground_1 : Ground
  Ground_2 : Ground
  ErrorCode : ArgOut  OutDataTypeStr=Enum: Dcm_NegativeResponseCodeType  ArgumentName=ErrorCode
  ERR : ArgOut  Port=2  OutDataTypeStr=Std_ReturnType  ArgumentName=ERR
  wires:
  Ground_1/1 -> ErrorCode/1
  Ground_2/1 -> ERR/1

== system cddDcmApp/DataServices_DID_D978_Stepper_Motor_16_Mode_Floor_First_Left_Flap_DID_D978_2_No_Of_Movements/DataServices_DID_D978_Stepper_Motor_16_Mode_Floor_First_Left_Flap_DID_D978_2_No_Of_Movements_ReadData_sys ==
  function : TriggerPort  TriggerType=function-call  IsSimulinkFunction=on  FunctionName=DataServices_DID_D978_Stepper_Motor_16_Mode_Floor_First_Left_Flap_DID_D978_2_No_Of_Movements_ReadData  VariantControl=(inherit)
  Ground_1 : Ground
  Ground_2 : Ground
  Data : ArgOut  OutDataTypeStr=uint8  ArgumentName=Data
  ERR : ArgOut  Port=2  OutDataTypeStr=Std_ReturnType  ArgumentName=ERR
  wires:
  Ground_1/1 -> Data/1
  Ground_2/1 -> ERR/1

== system cddDcmApp/DataServices_DID_D978_Stepper_Motor_16_Mode_Floor_First_Left_Flap_DID_D978_2_No_Of_Movements/DataServices_DID_D978_Stepper_Motor_16_Mode_Floor_First_Left_Flap_DID_D978_2_No_Of_Movements_WriteData_sys ==
  function : TriggerPort  TriggerType=function-call  IsSimulinkFunction=on  FunctionName=DataServices_DID_D978_Stepper_Motor_16_Mode_Floor_First_Left_Flap_DID_D978_2_No_Of_Movements_WriteData  VariantControl=(inherit)
  Ground_1 : Ground
  Ground_2 : Ground
  Terminator_1 : Terminator
  Terminator_2 : Terminator
  Data : ArgIn  OutDataTypeStr=uint8  PortDimensions=4  ArgumentName=Data
  OpStatus : ArgIn  Port=2  OutDataTypeStr=Enum: Dcm_OpStatusType  ArgumentName=OpStatus
  ErrorCode : ArgOut  OutDataTypeStr=Enum: Dcm_NegativeResponseCodeType  ArgumentName=ErrorCode
  ERR : ArgOut  Port=2  OutDataTypeStr=Std_ReturnType  ArgumentName=ERR
  wires:
  Data/1 -> Terminator_1/1
  OpStatus/1 -> Terminator_2/1
  Ground_1/1 -> ErrorCode/1
  Ground_2/1 -> ERR/1

== system cddDcmApp/DataServices_DID_D979_Stepper_Motor_16_Mode_Floor_First_Left_Flap_position_ComplexStructure_2/DataServices_DID_D979_Stepper_Motor_16_Mode_Floor_First_Left_Flap_position_ComplexStructure_2_ConditionCheckRead_sys ==
  function : TriggerPort  TriggerType=function-call  IsSimulinkFunction=on  FunctionName=DataServices_DID_D979_Stepper_Motor_16_Mode_Floor_First_Left_Flap_position_ComplexStructure_2_ConditionCheckRead  VariantControl=(inherit)
  Ground_1 : Ground
  Ground_2 : Ground
  ErrorCode : ArgOut  OutDataTypeStr=Enum: Dcm_NegativeResponseCodeType  ArgumentName=ErrorCode
  ERR : ArgOut  Port=2  OutDataTypeStr=Std_ReturnType  ArgumentName=ERR
  wires:
  Ground_1/1 -> ErrorCode/1
  Ground_2/1 -> ERR/1

== system cddDcmApp/DataServices_DID_D979_Stepper_Motor_16_Mode_Floor_First_Left_Flap_position_ComplexStructure_2/DataServices_DID_D979_Stepper_Motor_16_Mode_Floor_First_Left_Flap_position_ComplexStructure_2_ReadData_sys ==
  function : TriggerPort  TriggerType=function-call  IsSimulinkFunction=on  FunctionName=DataServices_DID_D979_Stepper_Motor_16_Mode_Floor_First_Left_Flap_position_ComplexStructure_2_ReadData  VariantControl=(inherit)
  Ground_1 : Ground
  Ground_2 : Ground
  Data : ArgOut  OutDataTypeStr=uint8  ArgumentName=Data
  ERR : ArgOut  Port=2  OutDataTypeStr=Std_ReturnType  ArgumentName=ERR
  wires:
  Ground_1/1 -> Data/1
  Ground_2/1 -> ERR/1

== system cddDcmApp/DataServices_DID_D979_Stepper_Motor_16_Mode_Floor_First_Left_Flap_position_DID_D979_1_Requested_Position/DataServices_DID_D979_Stepper_Motor_16_Mode_Floor_First_Left_Flap_position_DID_D979_1_Requested_Position_ConditionCheckRead_sys ==
  function : TriggerPort  TriggerType=function-call  IsSimulinkFunction=on  FunctionName=<binary blob, 123 chars, not shown>  VariantControl=(inherit)
  Ground_1 : Ground
  Ground_2 : Ground
  ErrorCode : ArgOut  OutDataTypeStr=Enum: Dcm_NegativeResponseCodeType  ArgumentName=ErrorCode
  ERR : ArgOut  Port=2  OutDataTypeStr=Std_ReturnType  ArgumentName=ERR
  wires:
  Ground_1/1 -> ErrorCode/1
  Ground_2/1 -> ERR/1

== system cddDcmApp/DataServices_DID_D979_Stepper_Motor_16_Mode_Floor_First_Left_Flap_position_DID_D979_1_Requested_Position/DataServices_DID_D979_Stepper_Motor_16_Mode_Floor_First_Left_Flap_position_DID_D979_1_Requested_Position_ReadData_sys ==
  function : TriggerPort  TriggerType=function-call  IsSimulinkFunction=on  FunctionName=DataServices_DID_D979_Stepper_Motor_16_Mode_Floor_First_Left_Flap_position_DID_D979_1_Requested_Position_ReadData  VariantControl=(inherit)
  Ground_1 : Ground
  Ground_2 : Ground
  Data : ArgOut  OutDataTypeStr=uint8  ArgumentName=Data
  ERR : ArgOut  Port=2  OutDataTypeStr=Std_ReturnType  ArgumentName=ERR
  wires:
  Ground_1/1 -> Data/1
  Ground_2/1 -> ERR/1

== system cddDcmApp/DataServices_DID_D979_Stepper_Motor_16_Mode_Floor_First_Left_Flap_position_DID_D979_2_Actual_Position/DataServices_DID_D979_Stepper_Motor_16_Mode_Floor_First_Left_Flap_position_DID_D979_2_Actual_Position_ConditionCheckRead_sys ==
  function : TriggerPort  TriggerType=function-call  IsSimulinkFunction=on  FunctionName=<binary blob, 120 chars, not shown>  VariantControl=(inherit)
  Ground_1 : Ground
  Ground_2 : Ground
  ErrorCode : ArgOut  OutDataTypeStr=Enum: Dcm_NegativeResponseCodeType  ArgumentName=ErrorCode
  ERR : ArgOut  Port=2  OutDataTypeStr=Std_ReturnType  ArgumentName=ERR
  wires:
  Ground_1/1 -> ErrorCode/1
  Ground_2/1 -> ERR/1

== system cddDcmApp/DataServices_DID_D979_Stepper_Motor_16_Mode_Floor_First_Left_Flap_position_DID_D979_2_Actual_Position/DataServices_DID_D979_Stepper_Motor_16_Mode_Floor_First_Left_Flap_position_DID_D979_2_Actual_Position_ReadData_sys ==
  function : TriggerPort  TriggerType=function-call  IsSimulinkFunction=on  FunctionName=DataServices_DID_D979_Stepper_Motor_16_Mode_Floor_First_Left_Flap_position_DID_D979_2_Actual_Position_ReadData  VariantControl=(inherit)
  Ground_1 : Ground
  Ground_2 : Ground
  Data : ArgOut  OutDataTypeStr=uint8  ArgumentName=Data
  ERR : ArgOut  Port=2  OutDataTypeStr=Std_ReturnType  ArgumentName=ERR
  wires:
  Ground_1/1 -> Data/1
  Ground_2/1 -> ERR/1

== system cddDcmApp/DataServices_DID_D97A_Stepper_Motor_17_Mode_Floor_First_Right_Flap_DID_D97A_1_step_Range/DataServices_DID_D97A_Stepper_Motor_17_Mode_Floor_First_Right_Flap_DID_D97A_1_step_Range_ConditionCheckRead_sys ==
  function : TriggerPort  TriggerType=function-call  IsSimulinkFunction=on  FunctionName=DataServices_DID_D97A_Stepper_Motor_17_Mode_Floor_First_Right_Flap_DID_D97A_1_step_Range_ConditionCheckRead  VariantControl=(inherit)
  Ground_1 : Ground
  Ground_2 : Ground
  ErrorCode : ArgOut  OutDataTypeStr=Enum: Dcm_NegativeResponseCodeType  ArgumentName=ErrorCode
  ERR : ArgOut  Port=2  OutDataTypeStr=Std_ReturnType  ArgumentName=ERR
  wires:
  Ground_1/1 -> ErrorCode/1
  Ground_2/1 -> ERR/1

== system cddDcmApp/DataServices_DID_D97A_Stepper_Motor_17_Mode_Floor_First_Right_Flap_DID_D97A_1_step_Range/DataServices_DID_D97A_Stepper_Motor_17_Mode_Floor_First_Right_Flap_DID_D97A_1_step_Range_ReadData_sys ==
  function : TriggerPort  TriggerType=function-call  IsSimulinkFunction=on  FunctionName=DataServices_DID_D97A_Stepper_Motor_17_Mode_Floor_First_Right_Flap_DID_D97A_1_step_Range_ReadData  VariantControl=(inherit)
  Ground_1 : Ground
  Ground_2 : Ground
  Data : ArgOut  OutDataTypeStr=uint8  ArgumentName=Data
  ERR : ArgOut  Port=2  OutDataTypeStr=Std_ReturnType  ArgumentName=ERR
  wires:
  Ground_1/1 -> Data/1
  Ground_2/1 -> ERR/1

== system cddDcmApp/DataServices_DID_D97A_Stepper_Motor_17_Mode_Floor_First_Right_Flap_DID_D97A_1_step_Range/DataServices_DID_D97A_Stepper_Motor_17_Mode_Floor_First_Right_Flap_DID_D97A_1_step_Range_WriteData_sys ==
  function : TriggerPort  TriggerType=function-call  IsSimulinkFunction=on  FunctionName=DataServices_DID_D97A_Stepper_Motor_17_Mode_Floor_First_Right_Flap_DID_D97A_1_step_Range_WriteData  VariantControl=(inherit)
  Ground_1 : Ground
  Ground_2 : Ground
  Terminator_1 : Terminator
  Terminator_2 : Terminator
  Data : ArgIn  OutDataTypeStr=uint8  PortDimensions=2  ArgumentName=Data
  OpStatus : ArgIn  Port=2  OutDataTypeStr=Enum: Dcm_OpStatusType  ArgumentName=OpStatus
  ErrorCode : ArgOut  OutDataTypeStr=Enum: Dcm_NegativeResponseCodeType  ArgumentName=ErrorCode
  ERR : ArgOut  Port=2  OutDataTypeStr=Std_ReturnType  ArgumentName=ERR
  wires:
  Data/1 -> Terminator_1/1
  OpStatus/1 -> Terminator_2/1
  Ground_1/1 -> ErrorCode/1
  Ground_2/1 -> ERR/1

== system cddDcmApp/DataServices_DID_D97A_Stepper_Motor_17_Mode_Floor_First_Right_Flap_DID_D97A_2_No_Of_Movements/DataServices_DID_D97A_Stepper_Motor_17_Mode_Floor_First_Right_Flap_DID_D97A_2_No_Of_Movements_ConditionCheckRead_sys ==
  function : TriggerPort  TriggerType=function-call  IsSimulinkFunction=on  FunctionName=DataServices_DID_D97A_Stepper_Motor_17_Mode_Floor_First_Right_Flap_DID_D97A_2_No_Of_Movements_ConditionCheckRead  VariantControl=(inherit)
  Ground_1 : Ground
  Ground_2 : Ground
  ErrorCode : ArgOut  OutDataTypeStr=Enum: Dcm_NegativeResponseCodeType  ArgumentName=ErrorCode
  ERR : ArgOut  Port=2  OutDataTypeStr=Std_ReturnType  ArgumentName=ERR
  wires:
  Ground_1/1 -> ErrorCode/1
  Ground_2/1 -> ERR/1

== system cddDcmApp/DataServices_DID_D97A_Stepper_Motor_17_Mode_Floor_First_Right_Flap_DID_D97A_2_No_Of_Movements/DataServices_DID_D97A_Stepper_Motor_17_Mode_Floor_First_Right_Flap_DID_D97A_2_No_Of_Movements_ReadData_sys ==
  function : TriggerPort  TriggerType=function-call  IsSimulinkFunction=on  FunctionName=DataServices_DID_D97A_Stepper_Motor_17_Mode_Floor_First_Right_Flap_DID_D97A_2_No_Of_Movements_ReadData  VariantControl=(inherit)
  Ground_1 : Ground
  Ground_2 : Ground
  Data : ArgOut  OutDataTypeStr=uint8  ArgumentName=Data
  ERR : ArgOut  Port=2  OutDataTypeStr=Std_ReturnType  ArgumentName=ERR
  wires:
  Ground_1/1 -> Data/1
  Ground_2/1 -> ERR/1

== system cddDcmApp/DataServices_DID_D97A_Stepper_Motor_17_Mode_Floor_First_Right_Flap_DID_D97A_2_No_Of_Movements/DataServices_DID_D97A_Stepper_Motor_17_Mode_Floor_First_Right_Flap_DID_D97A_2_No_Of_Movements_WriteData_sys ==
  function : TriggerPort  TriggerType=function-call  IsSimulinkFunction=on  FunctionName=DataServices_DID_D97A_Stepper_Motor_17_Mode_Floor_First_Right_Flap_DID_D97A_2_No_Of_Movements_WriteData  VariantControl=(inherit)
  Ground_1 : Ground
  Ground_2 : Ground
  Terminator_1 : Terminator
  Terminator_2 : Terminator
  Data : ArgIn  OutDataTypeStr=uint8  PortDimensions=4  ArgumentName=Data
  OpStatus : ArgIn  Port=2  OutDataTypeStr=Enum: Dcm_OpStatusType  ArgumentName=OpStatus
  ErrorCode : ArgOut  OutDataTypeStr=Enum: Dcm_NegativeResponseCodeType  ArgumentName=ErrorCode
  ERR : ArgOut  Port=2  OutDataTypeStr=Std_ReturnType  ArgumentName=ERR
  wires:
  Data/1 -> Terminator_1/1
  OpStatus/1 -> Terminator_2/1
  Ground_1/1 -> ErrorCode/1
  Ground_2/1 -> ERR/1

== system cddDcmApp/DataServices_DID_D97B_Stepper_Motor_17_Mode_Floor_First_Right_Flap_position_ComplexStructure_2/DataServices_DID_D97B_Stepper_Motor_17_Mode_Floor_First_Right_Flap_position_ComplexStructure_2_ConditionCheckRead_sys ==
  function : TriggerPort  TriggerType=function-call  IsSimulinkFunction=on  FunctionName=DataServices_DID_D97B_Stepper_Motor_17_Mode_Floor_First_Right_Flap_position_ComplexStructure_2_ConditionCheckRead  VariantControl=(inherit)
  Ground_1 : Ground
  Ground_2 : Ground
  ErrorCode : ArgOut  OutDataTypeStr=Enum: Dcm_NegativeResponseCodeType  ArgumentName=ErrorCode
  ERR : ArgOut  Port=2  OutDataTypeStr=Std_ReturnType  ArgumentName=ERR
  wires:
  Ground_1/1 -> ErrorCode/1
  Ground_2/1 -> ERR/1

== system cddDcmApp/DataServices_DID_D97B_Stepper_Motor_17_Mode_Floor_First_Right_Flap_position_ComplexStructure_2/DataServices_DID_D97B_Stepper_Motor_17_Mode_Floor_First_Right_Flap_position_ComplexStructure_2_ReadData_sys ==
  function : TriggerPort  TriggerType=function-call  IsSimulinkFunction=on  FunctionName=DataServices_DID_D97B_Stepper_Motor_17_Mode_Floor_First_Right_Flap_position_ComplexStructure_2_ReadData  VariantControl=(inherit)
  Ground_1 : Ground
  Ground_2 : Ground
  Data : ArgOut  OutDataTypeStr=uint8  ArgumentName=Data
  ERR : ArgOut  Port=2  OutDataTypeStr=Std_ReturnType  ArgumentName=ERR
  wires:
  Ground_1/1 -> Data/1
  Ground_2/1 -> ERR/1

== system cddDcmApp/DataServices_DID_D97B_Stepper_Motor_17_Mode_Floor_First_Right_Flap_position_DID_D97B_1_Requested_Position/DataServices_DID_D97B_Stepper_Motor_17_Mode_Floor_First_Right_Flap_position_DID_D97B_1_Requested_Position_ConditionCheckRead_sys ==
  function : TriggerPort  TriggerType=function-call  IsSimulinkFunction=on  FunctionName=<binary blob, 124 chars, not shown>  VariantControl=(inherit)
  Ground_1 : Ground
  Ground_2 : Ground
  ErrorCode : ArgOut  OutDataTypeStr=Enum: Dcm_NegativeResponseCodeType  ArgumentName=ErrorCode
  ERR : ArgOut  Port=2  OutDataTypeStr=Std_ReturnType  ArgumentName=ERR
  wires:
  Ground_1/1 -> ErrorCode/1
  Ground_2/1 -> ERR/1
